simulink model "reduce_spect"  (R2013b) (continued)
== system reduce_spect == (continued)
      mask prompts: storage "Storage medium"; dram_dimm "DRAM dimm"; dram_clock "DRAM clock rate"; nsamples "Number of Samples (2^?)"; data_width "Data width"; offset "Start delay support"; circap "Circular capture support"; value "Extra value capture support"; use_dsp48 "Use DSP48s to implement counters"
      mask help:
      | Standard snap block, but with the following optional extras;
      |  1) Optionally delay the fabric trigger by a user-specified number of valids.
      |  2) Capture continuously after a trigger until a stop pulse is received. Data is captured into BRAM continuously, with old data being overwritten until a stop command is received. Then stop capturing when memory is filled. This enables you to get some data before and some data after the stop pulse. Use the 'tr_en_cnt' register to determine where you are in your vector. 'Addr' register's MSb now indicates 'busy capturing'.
      |  3) Optionally capture a value on an input port to a register at the same time as the first data sample.
      |  4) Implement counters using DSP48Es to save resources.
      mask init: same as reduce_spect/adcsnap1
      mask TypeOptions storage: bram | dram
      mask Callback storage: snapshot_callback();
      mask TypeOptions dram_dimm: 1 | 2 | 3 | 4
      mask TypeOptions dram_clock: 200 | 250 | 266 | 300 | 333
      mask TypeOptions data_width: 8 | 16 | 32 | 64 | 128
  spec1_0 : Subsystem  Tag=xps:bram  AncestorBlock=xps_library/Shared_BRAM  mask{arith_type=Unsigned, addr_width=9, data_width=64, reg_prim_output=on, reg_core_output=on, optimization=Minimum_Area, data_bin_pt=0, init_vals=[0:2^10-1], sample_rate=1}
      mask prompts: arith_type "Output Data Type"; addr_width "Address width"; data_width "Data Width"; reg_prim_output "Register Primitive Output"; reg_core_output "Register Core Output"; data_bin_pt "Data Binary Point"; init_vals "Initial values (simulation only)"; sample_rate "Sample rate"
      mask init:
      | shared_bram_mask;
      mask TypeOptions arith_type: Unsigned | Signed  (2's comp)
      mask TypeOptions data_width: 8 | 16 | 32 | 64 | 128
      mask TypeOptions optimization: Minimum_Area | Low_Power
  spec1_1 : Subsystem  Tag=xps:bram  AncestorBlock=xps_library/Shared_BRAM  mask{arith_type=Unsigned, addr_width=9, data_width=64, reg_prim_output=on, reg_core_output=on, optimization=Minimum_Area, data_bin_pt=0, init_vals=[0:2^10-1], sample_rate=1}
      mask prompts: arith_type "Output Data Type"; addr_width "Address width"; data_width "Data Width"; reg_prim_output "Register Primitive Output"; reg_core_output "Register Core Output"; data_bin_pt "Data Binary Point"; init_vals "Initial values (simulation only)"; sample_rate "Sample rate"
      mask init:
      | shared_bram_mask;
      mask TypeOptions arith_type: Unsigned | Signed  (2's comp)
      mask TypeOptions data_width: 8 | 16 | 32 | 64 | 128
      mask TypeOptions optimization: Minimum_Area | Low_Power
  spec1_2 : Subsystem  Tag=xps:bram  AncestorBlock=xps_library/Shared_BRAM  mask{arith_type=Unsigned, addr_width=9, data_width=64, reg_prim_output=on, reg_core_output=on, optimization=Minimum_Area, data_bin_pt=0, init_vals=[0:2^10-1], sample_rate=1}
      mask prompts: arith_type "Output Data Type"; addr_width "Address width"; data_width "Data Width"; reg_prim_output "Register Primitive Output"; reg_core_output "Register Core Output"; data_bin_pt "Data Binary Point"; init_vals "Initial values (simulation only)"; sample_rate "Sample rate"
      mask init:
      | shared_bram_mask;
      mask TypeOptions arith_type: Unsigned | Signed  (2's comp)
      mask TypeOptions data_width: 8 | 16 | 32 | 64 | 128
      mask TypeOptions optimization: Minimum_Area | Low_Power
  spec1_3 : Subsystem  Tag=xps:bram  AncestorBlock=xps_library/Shared_BRAM  mask{arith_type=Unsigned, addr_width=9, data_width=64, reg_prim_output=on, reg_core_output=on, optimization=Minimum_Area, data_bin_pt=0, init_vals=[0:2^10-1], sample_rate=1}
      mask prompts: arith_type "Output Data Type"; addr_width "Address width"; data_width "Data Width"; reg_prim_output "Register Primitive Output"; reg_core_output "Register Core Output"; data_bin_pt "Data Binary Point"; init_vals "Initial values (simulation only)"; sample_rate "Sample rate"
      mask init:
      | shared_bram_mask;
      mask TypeOptions arith_type: Unsigned | Signed  (2's comp)
      mask TypeOptions data_width: 8 | 16 | 32 | 64 | 128
      mask TypeOptions optimization: Minimum_Area | Low_Power
  spec2_0 : Subsystem  Tag=xps:bram  AncestorBlock=xps_library/Shared_BRAM  mask{arith_type=Unsigned, addr_width=9, data_width=64, reg_prim_output=on, reg_core_output=on, optimization=Minimum_Area, data_bin_pt=0, init_vals=[0:2^10-1], sample_rate=1}
      mask prompts: arith_type "Output Data Type"; addr_width "Address width"; data_width "Data Width"; reg_prim_output "Register Primitive Output"; reg_core_output "Register Core Output"; data_bin_pt "Data Binary Point"; init_vals "Initial values (simulation only)"; sample_rate "Sample rate"
      mask init:
      | shared_bram_mask;
      mask TypeOptions arith_type: Unsigned | Signed  (2's comp)
      mask TypeOptions data_width: 8 | 16 | 32 | 64 | 128
      mask TypeOptions optimization: Minimum_Area | Low_Power
  spec2_1 : Subsystem  Tag=xps:bram  AncestorBlock=xps_library/Shared_BRAM  mask{arith_type=Unsigned, addr_width=9, data_width=64, reg_prim_output=on, reg_core_output=on, optimization=Minimum_Area, data_bin_pt=0, init_vals=[0:2^10-1], sample_rate=1}
      mask prompts: arith_type "Output Data Type"; addr_width "Address width"; data_width "Data Width"; reg_prim_output "Register Primitive Output"; reg_core_output "Register Core Output"; data_bin_pt "Data Binary Point"; init_vals "Initial values (simulation only)"; sample_rate "Sample rate"
      mask init:
      | shared_bram_mask;
      mask TypeOptions arith_type: Unsigned | Signed  (2's comp)
      mask TypeOptions data_width: 8 | 16 | 32 | 64 | 128
      mask TypeOptions optimization: Minimum_Area | Low_Power
  spec2_2 : Subsystem  Tag=xps:bram  AncestorBlock=xps_library/Shared_BRAM  mask{arith_type=Unsigned, addr_width=9, data_width=64, reg_prim_output=on, reg_core_output=on, optimization=Minimum_Area, data_bin_pt=0, init_vals=[0:2^10-1], sample_rate=1}
      mask prompts: arith_type "Output Data Type"; addr_width "Address width"; data_width "Data Width"; reg_prim_output "Register Primitive Output"; reg_core_output "Register Core Output"; data_bin_pt "Data Binary Point"; init_vals "Initial values (simulation only)"; sample_rate "Sample rate"
      mask init:
      | shared_bram_mask;
      mask TypeOptions arith_type: Unsigned | Signed  (2's comp)
      mask TypeOptions data_width: 8 | 16 | 32 | 64 | 128
      mask TypeOptions optimization: Minimum_Area | Low_Power
  spec2_3 : Subsystem  Tag=xps:bram  AncestorBlock=xps_library/Shared_BRAM  mask{arith_type=Unsigned, addr_width=9, data_width=64, reg_prim_output=on, reg_core_output=on, optimization=Minimum_Area, data_bin_pt=0, init_vals=[0:2^10-1], sample_rate=1}
      mask prompts: arith_type "Output Data Type"; addr_width "Address width"; data_width "Data Width"; reg_prim_output "Register Primitive Output"; reg_core_output "Register Core Output"; data_bin_pt "Data Binary Point"; init_vals "Initial values (simulation only)"; sample_rate "Sample rate"
      mask init:
      | shared_bram_mask;
      mask TypeOptions arith_type: Unsigned | Signed  (2's comp)
      mask TypeOptions data_width: 8 | 16 | 32 | 64 | 128
      mask TypeOptions optimization: Minimum_Area | Low_Power
  sync_gen : Subsystem  AncestorBlock=casper_library_misc/sync_gen  mask(sync_gen){sim_acc_len=1, fft_size=2048, fft_simult_inputs=8, pfb_fir_taps=4, reorder_vec=[2 2 9], scale=1}
      mask prompts: sim_acc_len "Simulation Accumulation Length"; fft_size "FFT Size"; fft_simult_inputs "Simultaneous Inputs (FFT)"; pfb_fir_taps "Taps in pfb_fir (use 1 if no pfb_fir)"; reorder_vec "Reorder Orders (e.g. [2 2 2])"
      mask help:
      | This block computes the sync period based on system parameters as
      | described in Casper memo.  Detailed descritions of the block's
      | variables can be found in the 'Block Documentation' section of
      | the CASPER wiki.
      mask init:
      | sync_gen_init(gcb, ...
      |     'sim_acc_len', sim_acc_len, ...
      |     'fft_size', fft_size, ...
      |     'fft_simult_inputs', fft_simult_inputs,...
      |     'pfb_fir_taps', pfb_fir_taps, ...
      |     'reorder_vec', reorder_vec, ...
      |     'scale', scale);
      settings: AttributesFormatString=sim_sync_period=18432
      mask Help:
      | SyncPeriod=LCM(reorder_vec)*(acc_num)*(pfb_fir_len)*(fft_size/fft_simult_inputs)
      | 
      | See CASPER sync pulse memo for more details.
  sync_tree2 : Subsystem
  ten_Gbe_v2 : Subsystem  Tag=xps:tengbe_v2  AncestorBlock=xps_library/ten_Gbe_v2  mask(ten_GbE_v2){flavour=sfp+, slot=0, port_r1=0, port_r2_cx4=0, port_r2_sfpp=0, rx_dist_ram=off, large_frames=on, show_param=on, pre_emph_r2=1.07, pre_emph=3, post_emph_r2=0.18, rxeqmix_r2=7, swing_r2=940, swing=800, fab_en=on, fab_mac=hex2dec('123456780000'), fab_ip=192*(2^24) + 168*(2^16) + 5*(2^8) + 20*(2^0), fab_udp=10000, fab_gate=1, cpu_rx_en=off, cpu_tx_en=on, ttl=hex2dec('FF'), promisc_mode=off, debug_ctr_width=16, txctr=on, txerrctr=on, txerrctr_len=100, txofctr=on, txfullctr=on, txvldctr=on, txsnaplen=16384, rxctr=off, rxerrctr=off, rxerrctr_len=100, rxofctr=off, rxbadctr=off, rxvldctr=off, rxeofctr=off, rxsnaplen=0 - no snap}
      mask prompts: flavour "Mezzanine card flavour"; slot "Card slot"; port_r1 "Port"; port_r2_cx4 "Port"; port_r2_sfpp "Port"; rx_dist_ram "Shallow RX Fifo (Beware overruns!)"; large_frames "Enable Large TX Frames (8k+512)"; show_param "---------------------- Show Implementation Parameters -----------------------------------------------------------------------------------"; pre_emph_r2 "Pre-emphasis (dB)"; pre_emph "Pre-emphasis"; post_emph_r2 "Post-emphasis (dB)"; rxeqmix_r2 "Receive equalisation"; swing_r2 "Driver Swing Control (mVppd)"; swing "Differential swing"; fab_en "Enable fabric on startup"; fab_mac "Fabric MAC Address"; fab_ip "Fabric IP Address"; fab_udp "Fabric UDP Port"; fab_gate "Fabric Gateway"; cpu_rx_en "Enable CPU RX"; cpu_tx_en "Enable CPU TX"; promisc_mode "Promiscuous mode"; debug_ctr_width "Debug counter width"; txctr "TX - core_txctr"; txerrctr "TX packet length error - core_txerrctr"; txerrctr_len "-- expected TX packet length"; txofctr "TX overflow - core_txofctr"; txfullctr "TX full - core_txfullctr"; txvldctr "TX valid cycle - core_txvldctr"; txsnaplen "TX snapshot length"; rxctr "RX - core_rxctr"; rxerrctr "RX packet length error - core_rxerrctr"; rxerrctr_len "-- expected RX packet length"; rxofctr "RX overflow - core_rxofctr"; rxbadctr "RX bad - core_rxbadctr"; rxvldctr "RX valid cycle - core_rxvldctr"; rxeofctr "RX eof - core_rxeofctr"; rxsnaplen "RX snapshot length"
      mask help: This block sends and receives UDP frames (packets). It accepts a 64 bit wide data stream with user-determined frame breaks. The data stream is wrapped in a UDP frame for transmission. Incoming UDP packets are unwrapped and the data presented as a 64 bit wide stream.
      mask init:
      | temp = eval(get_param(gcb, 'debug_ctr_width'));
      | if temp <= 0,
      |     error('Debug counters cannot be ltoet zero.');
      | end
      | tengbe_v2_mask(gcb)
      mask TypeOptions flavour: sfp+ | cx4
      mask Callback flavour: tengbe_v2_callback
      mask TypeOptions slot: 0 | 1
      mask TypeOptions port_r1: 0 | 1 | 2 | 3
      mask TypeOptions port_r2_cx4: 0 | 1 | 2
      mask TypeOptions port_r2_sfpp: 0 | 1 | 2 | 3
      mask Callback show_param: tengbe_v2_callback
      mask TypeOptions pre_emph_r2: 0.15 | 0.3 | 0.45 | 0.61 | 0.74 | 0.91 | 1.07 | 1.25 | 1.36 | 1.55 | 1.74 | 1.94 | 2.11 | 2.32 | 2.54 | 2.77
      mask TypeOptions pre_emph: 0 | 1 | 2 | 3
      mask TypeOptions post_emph_r2: 0.18 | 0.19 | 0.2 | 0.39 | 0.63 | 0.82 | 1.07 | 1.32 | 1.6 | 1.65 | 1.94 | 2.21 | 2.52 | 2.76 | 3.08 | 3.41 | 3.77 | 3.97 | 4.36 | 4.73 | 5.16 | 5.47 | 5.93 | 6.38 | 6.89
      mask TypeOptions rxeqmix_r2: 0 | 1 | 2 | 3 | 4 | 5 | 6 | 7
      mask TypeOptions swing_r2: 110 | 210 | 310 | 400 | 480 | 570 | 660 | 740 | 810 | 880 | 940 | 990 | 1040 | 1080 | 1110 | 1130
      mask TypeOptions swing: 400 | 500 | 600 | 700 | 800
      mask Callback txerrctr:
      | blk = gcb;
      | mask_visibilities = get_param(blk, 'MaskVisibilities');
      | mask_names = get_param(blk, 'MaskNames');
      | if strcmp(get_param(blk, 'txerrctr'), 'on')
      |     mask_visibilities{ismember(mask_names, 'txerrctr_len')} = 'on';
      | else
      |     mask_visibilities{ismember(mask_names, 'txerrctr_len')} = 'off';
      | end
      | set_param(blk, 'MaskVisibilities', mask_visibilities);
      mask TypeOptions txsnaplen: 0 - no snap | 256 | 512 | 1024 | 2048 | 4096 | 8192 | 16384
      mask Callback rxerrctr:
      | blk = gcb;
      | mask_visibilities = get_param(blk, 'MaskVisibilities');
      | mask_names = get_param(blk, 'MaskNames');
      | if strcmp(get_param(blk, 'rxerrctr'), 'on')
      |     mask_visibilities{ismember(mask_names, 'rxerrctr_len')} = 'on';
      | else
      |     mask_visibilities{ismember(mask_names, 'rxerrctr_len')} = 'off';
      | end
      | set_param(blk, 'MaskVisibilities', mask_visibilities);
      mask TypeOptions rxsnaplen: 0 - no snap | 256 | 512 | 1024 | 2048 | 4096 | 8192 | 16384
  test : Subsystem  Tag=xps:bram  AncestorBlock=xps_library/Shared_BRAM  mask{arith_type=Unsigned, addr_width=10, data_width=32, reg_prim_output=off, reg_core_output=off, optimization=Minimum_Area, data_bin_pt=0, init_vals=[0:2^10-1], sample_rate=1}
      mask prompts: arith_type "Output Data Type"; addr_width "Address width"; data_width "Data Width"; reg_prim_output "Register Primitive Output"; reg_core_output "Register Core Output"; data_bin_pt "Data Binary Point"; init_vals "Initial values (simulation only)"; sample_rate "Sample rate"
      mask init:
      | shared_bram_mask;
      mask TypeOptions arith_type: Unsigned | Signed  (2's comp)
      mask TypeOptions data_width: 8 | 16 | 32 | 64 | 128
      mask TypeOptions optimization: Minimum_Area | Low_Power
  theta0 : Subsystem  Tag=xps:sw_reg  AncestorBlock=xps_library/software_register  mask(swreg){io_dir=From Processor, io_delay=0, sample_period=1, names=reg, bitwidths=32, bin_pts=0, arith_types=0, sim_port=off, show_format=on}
      mask prompts: io_dir "I/O direction"; io_delay "I/O delay"; sample_period "Sample period"; names "Bitfield names [msb...lsb]"; bitwidths "Bitfield widths"; bin_pts "Bitfield binary pts"; arith_types "Bitfield types, ufix=0, fix=1, bool=2"; sim_port "Provide sim input/output?"; show_format "Print format string?"
      mask help: A 32-bit software-accessible register. Can be divided into bitfields of varying widths and types using the fields in the block mask.
      mask init:
      | try
      |     swreg_init(gcb)
      | catch ex
      |     disp(sprintf('%s: %s', gcb, ex.message))
      |     errordlg(ex.message)
      | end
      settings: AttributesFormatString=1input:uf32.0 = 32 bits
      mask TypeOptions io_dir: To Processor | From Processor
      mask Callback io_dir:
      | mask_enables = get_param(gcb, 'MaskEnables');
      | mask_names = get_param(gcb, 'MaskNames');
      | if strcmp(get_param(gcb, 'io_dir'), 'To Processor'),
      |     mask_enables{ismember(mask_names, 'sample_period')} = 'off';
      | else
      |     mask_enables{ismember(mask_names, 'sample_period')} = 'on';
      | end
      | set_param(gcb, 'MaskEnables', mask_enables);
  theta1 : Subsystem  Tag=xps:sw_reg  AncestorBlock=xps_library/software_register  mask(swreg){io_dir=From Processor, io_delay=0, sample_period=1, names=reg, bitwidths=32, bin_pts=0, arith_types=0, sim_port=off, show_format=on}
      mask prompts: io_dir "I/O direction"; io_delay "I/O delay"; sample_period "Sample period"; names "Bitfield names [msb...lsb]"; bitwidths "Bitfield widths"; bin_pts "Bitfield binary pts"; arith_types "Bitfield types, ufix=0, fix=1, bool=2"; sim_port "Provide sim input/output?"; show_format "Print format string?"
      mask help: A 32-bit software-accessible register. Can be divided into bitfields of varying widths and types using the fields in the block mask.
      mask init:
      | try
      |     swreg_init(gcb)
      | catch ex
      |     disp(sprintf('%s: %s', gcb, ex.message))
      |     errordlg(ex.message)
      | end
      settings: AttributesFormatString=1input:uf32.0 = 32 bits
      mask TypeOptions io_dir: To Processor | From Processor
      mask Callback io_dir:
      | mask_enables = get_param(gcb, 'MaskEnables');
      | mask_names = get_param(gcb, 'MaskNames');
      | if strcmp(get_param(gcb, 'io_dir'), 'To Processor'),
      |     mask_enables{ismember(mask_names, 'sample_period')} = 'off';
      | else
      |     mask_enables{ismember(mask_names, 'sample_period')} = 'on';
      | end
      | set_param(gcb, 'MaskEnables', mask_enables);
  theta2 : Subsystem  Tag=xps:sw_reg  AncestorBlock=xps_library/software_register  mask(swreg){io_dir=From Processor, io_delay=0, sample_period=1, names=reg, bitwidths=32, bin_pts=0, arith_types=0, sim_port=off, show_format=on}
      mask prompts: io_dir "I/O direction"; io_delay "I/O delay"; sample_period "Sample period"; names "Bitfield names [msb...lsb]"; bitwidths "Bitfield widths"; bin_pts "Bitfield binary pts"; arith_types "Bitfield types, ufix=0, fix=1, bool=2"; sim_port "Provide sim input/output?"; show_format "Print format string?"
      mask help: A 32-bit software-accessible register. Can be divided into bitfields of varying widths and types using the fields in the block mask.
      mask init:
      | try
      |     swreg_init(gcb)
      | catch ex
      |     disp(sprintf('%s: %s', gcb, ex.message))
      |     errordlg(ex.message)
      | end
      settings: AttributesFormatString=1input:uf32.0 = 32 bits
      mask TypeOptions io_dir: To Processor | From Processor
      mask Callback io_dir:
      | mask_enables = get_param(gcb, 'MaskEnables');
      | mask_names = get_param(gcb, 'MaskNames');
      | if strcmp(get_param(gcb, 'io_dir'), 'To Processor'),
      |     mask_enables{ismember(mask_names, 'sample_period')} = 'off';
      | else
      |     mask_enables{ismember(mask_names, 'sample_period')} = 'on';
      | end
      | set_param(gcb, 'MaskEnables', mask_enables);
  theta3 : Subsystem  Tag=xps:sw_reg  AncestorBlock=xps_library/software_register  mask(swreg){io_dir=From Processor, io_delay=0, sample_period=1, names=reg, bitwidths=32, bin_pts=0, arith_types=0, sim_port=off, show_format=on}
      mask prompts: io_dir "I/O direction"; io_delay "I/O delay"; sample_period "Sample period"; names "Bitfield names [msb...lsb]"; bitwidths "Bitfield widths"; bin_pts "Bitfield binary pts"; arith_types "Bitfield types, ufix=0, fix=1, bool=2"; sim_port "Provide sim input/output?"; show_format "Print format string?"
      mask help: A 32-bit software-accessible register. Can be divided into bitfields of varying widths and types using the fields in the block mask.
      mask init:
      | try
      |     swreg_init(gcb)
      | catch ex
      |     disp(sprintf('%s: %s', gcb, ex.message))
      |     errordlg(ex.message)
      | end
      settings: AttributesFormatString=1input:uf32.0 = 32 bits
      mask TypeOptions io_dir: To Processor | From Processor
      mask Callback io_dir:
      | mask_enables = get_param(gcb, 'MaskEnables');
      | mask_names = get_param(gcb, 'MaskNames');
      | if strcmp(get_param(gcb, 'io_dir'), 'To Processor'),
      |     mask_enables{ismember(mask_names, 'sample_period')} = 'off';
      | else
      |     mask_enables{ismember(mask_names, 'sample_period')} = 'on';
      | end
      | set_param(gcb, 'MaskEnables', mask_enables);
  wires:
  cnt_rst/1(in_reg) -> pipeline5/1(d)
  pipeline5/1(q) -> Slice3/1, Slice/1
  Slice/1 -> [cnt_rst]
  acc_len/1(in_reg) -> pipeline6/1(d)
  pipeline6/1(q) -> [acc_len]
  sync_gen/1(sync_out) -> pipeline143/1(d)
  pipeline143/1(q) -> [sync_gen]
  [acc_len0] -> acc_cntrl2/1(acc_len)
  [cnt_rst] -> acc_cntrl2/3(rst)
  [sd0] -> acc_cntrl2/2(sync)
  [m0d0] -> Delay26/1
  [m0e0] -> Delay27/1
  Delay26/1 -> Delay28/1
  acc_cntrl2/1(new_acc) -> Delay29/1
  Delay27/1 -> Delay30/1
  Delay29/1 -> pipeline9/1(d), simple_bram_vacc/1(new_acc), Delay14/1
  Delay30/1 -> simple_bram_vacc/3(val_in)
  Register/1 -> [frb_detector_0]
  [rst_detect] -> Register/2
  Relational/1 -> Register/3, Register/1
  Accumulator1/1 -> Reinterpret3/1
  Counter2/1 -> ACC0/1(addr)
  ACC0/1(data_out) -> Terminator22/1
  theta0/1(in_reg) -> Convert1/1
  Convert1/1 -> Relational/1
  acc_len1/1(in_reg) -> pipeline30/1(d)
  pipeline30/1(q) -> [acc_len1]
  gain/1(in_reg) -> pipeline31/1(d)
  pipeline31/1(q) -> [gain]
  [sync_gen] -> pipeline41/1(d)
  Delay37/1 -> Delay57/1
  Delay35/1 -> power4/1
  pipeline41/1(q) -> pfb_fir_real/1(sync)
  asiaa_adc5g/17(sync_out) -> Terminator6/1
  conv_bus1/1(q0) -> pipeline301/1
  conv_bus1/2(q1) -> pipeline349/1
  conv_bus1/3(q2) -> pipeline399/1
  conv_bus1/4(q3) -> pipeline400/1
  conv_bus1/5(q4) -> pipeline401/1
  conv_bus1/6(q5) -> pipeline402/1
  conv_bus1/7(q6) -> pipeline300/1
  conv_bus1/8(q7) -> pipeline299/1
  pipeline34/1(q) -> pfb_fir_real/4(pol1_in3)
  pipeline37/1(q) -> pfb_fir_real/6(pol1_in5)
  pipeline32/1(q) -> pfb_fir_real/2(pol1_in1)
  pipeline33/1(q) -> pfb_fir_real/3(pol1_in2)
  pipeline36/1(q) -> pfb_fir_real/5(pol1_in4)
  pipeline38/1(q) -> pfb_fir_real/7(pol1_in6)
  pipeline39/1(q) -> pfb_fir_real/8(pol1_in7)
  pipeline40/1(q) -> pfb_fir_real/9(pol1_in8)
  Delay62/1 -> fft_wideband_real/1(sync)
  pfb_fir_real/1(sync_out) -> Delay62/1
  Constant/1 -> fft_wideband_real/2(shift)
  pfb_fir_real/2(pol1_out1) -> Delay32/1
  pfb_fir_real/3(pol1_out2) -> Delay61/1
  pfb_fir_real/4(pol1_out3) -> Delay63/1
  pfb_fir_real/5(pol1_out4) -> Delay79/1
  pfb_fir_real/6(pol1_out5) -> Delay34/1
  pfb_fir_real/7(pol1_out6) -> Delay38/1
  pfb_fir_real/8(pol1_out7) -> Delay39/1
  pfb_fir_real/9(pol1_out8) -> Delay48/1
  Delay48/1 -> fft_wideband_real/10(in07)
  Delay34/1 -> fft_wideband_real/7(in04)
  Delay79/1 -> fft_wideband_real/6(in03)
  Delay38/1 -> fft_wideband_real/8(in05)
  Delay63/1 -> fft_wideband_real/5(in02)
  Delay32/1 -> fft_wideband_real/3(in00)
  Delay39/1 -> fft_wideband_real/9(in06)
  Delay61/1 -> fft_wideband_real/4(in01)
  fft_wideband_real/6(of) -> Terminator7/1
  fft_wideband_real/1(sync_out) -> Delay37/1
  Delay58/1 -> power5/1
  Delay59/1 -> power6/1
  Delay60/1 -> power7/1
  fft_wideband_real/2(out00) -> Delay35/1
  fft_wideband_real/3(out01) -> Delay58/1
  fft_wideband_real/4(out02) -> Delay59/1
  fft_wideband_real/5(out03) -> Delay60/1
  bus_create1/1(bus_out) -> pipeline599/1(d)
  Constant123/1 -> adcsnap1/3(trig), adcsnap1/2(we)
  pipeline599/1(q) -> adcsnap1/1(din)
  Delay57/1 -> Delay68/1
  power7/1 -> Delay71/1
  power5/1 -> Delay69/1
  power4/1 -> Delay67/1
  power6/1 -> Delay70/1
  Delay71/1 -> pipeline347/1(d), Delay65/1
  Delay69/1 -> pipeline345/1(d), Delay73/1
  Delay66/1 -> acc_cntrl11/2(sync)
  [acc_len] -> acc_cntrl11/1(acc_len)
  [cnt_rst] -> acc_cntrl11/3(rst)
  Delay72/1 -> pipeline304/1(d)
  pipeline315/1(q) -> simple_bram_vacc46/1(new_acc)
  pipeline311/1(q) -> simple_bram_vacc46/2(din)
  pipeline314/1(q) -> pipeline105/1(d)
  acc_cntrl11/1(new_acc) -> pipeline398/1(d)
  pipeline304/1(q) -> pipeline394/1(d)
  simple_bram_vacc46/1(dout) -> pipeline97/1(d)
  spec1_0/1(data_out) -> Terminator46/1
  simple_bram_vacc46/2(valid) -> pipeline98/1(d)
  pipeline305/1(q) -> simple_bram_vacc43/1(new_acc)
  pipeline303/1(q) -> simple_bram_vacc43/2(din)
  simple_bram_vacc43/1(dout) -> pipeline99/1(d)
  spec1_1/1(data_out) -> Terminator43/1
  simple_bram_vacc43/2(valid) -> pipeline100/1(d)
  pipeline309/1(q) -> simple_bram_vacc45/1(new_acc)
  pipeline308/1(q) -> simple_bram_vacc45/2(din)
  simple_bram_vacc45/1(dout) -> pipeline101/1(d)
  spec1_2/1(data_out) -> Terminator45/1
  simple_bram_vacc45/2(valid) -> pipeline102/1(d)
  pipeline307/1(q) -> simple_bram_vacc44/1(new_acc)
  pipeline306/1(q) -> simple_bram_vacc44/2(din)
  simple_bram_vacc44/1(dout) -> pipeline103/1(d)
  spec1_3/1(data_out) -> Terminator44/1
  simple_bram_vacc44/2(valid) -> pipeline104/1(d)
  Counter22/1 -> spec1_0/1(addr), spec1_1/1(addr), spec1_2/1(addr), spec1_3/1(addr)
  Delay73/1 -> pipeline310/1(d)
  pipeline310/1(q) -> pipeline395/1(d)
  Delay64/1 -> pipeline312/1(d)
  pipeline312/1(q) -> pipeline396/1(d)
  Delay65/1 -> pipeline313/1(d)
  pipeline313/1(q) -> pipeline397/1(d)
  Delay68/1 -> pipeline351/1(d), Delay66/1
  Delay67/1 -> pipeline348/1(d), Delay72/1
  Delay70/1 -> pipeline346/1(d), Delay64/1
  pipeline397/1(q) -> pipeline306/1(d)
  pipeline394/1(q) -> pipeline311/1(d)
  pipeline396/1(q) -> pipeline308/1(d)
  pipeline395/1(q) -> pipeline303/1(d)
  pipeline398/1(q) -> pipeline314/1(d), pipeline307/1(d), pipeline309/1(d), pipeline305/1(d), pipeline315/1(d)
  pipeline298/1 -> bus_expand/1(bus_in)
  conv_bus1/9(q8) -> pipeline298/1, [adc1_a_red], [adc0_a_red]
  pipeline299/1 -> pipeline40/1(d), bus_create1/8(in8)
  pipeline300/1 -> pipeline39/1(d), bus_create1/7(in7)
  pipeline301/1 -> pipeline32/1(d), bus_create1/1(in1)
  pipeline349/1 -> pipeline33/1(d), bus_create1/2(in2)
  pipeline399/1 -> pipeline34/1(d), bus_create1/3(in3)
  pipeline400/1 -> pipeline36/1(d), bus_create1/4(in4)
  pipeline401/1 -> pipeline37/1(d), bus_create1/5(in5)
  pipeline402/1 -> pipeline38/1(d), bus_create1/6(in6)
  pipeline351/1(q) -> adder_tree1/1(sync)
  pipeline348/1(q) -> adder_tree1/2(din1)
  pipeline345/1(q) -> adder_tree1/3(din2)
  pipeline346/1(q) -> adder_tree1/4(din3)
  pipeline347/1(q) -> adder_tree1/5(din4)
  Counter4/1 -> Slice8/1, Slice7/1, Slice6/1
  Slice6/1 -> Logical1/2
  Slice7/1 -> Logical1/3
  Subsystem3/1(Out1) -> Delay7/1
  [gain] -> Mult5/2
  Mult5/1 -> pipeline15/1(d)
  pipeline49/1(q) -> pipeline16/1(d)
  Delay82/1 -> Delay/1
  Slice8/1 -> Logical1/1
  asiaa_adc5g/6(a5) -> conv_bus1/7(i5)
  asiaa_adc5g/4(a3) -> conv_bus1/5(i3)
  asiaa_adc5g/1(a0) -> conv_bus1/2(i0)
  asiaa_adc5g/5(a4) -> conv_bus1/6(i4)
  asiaa_adc5g/2(a1) -> conv_bus1/3(i1)
  asiaa_adc5g/3(a2) -> conv_bus1/4(i2)
  asiaa_adc5g/7(a6) -> conv_bus1/8(i6)
  asiaa_adc5g/8(a7) -> conv_bus1/9(i7)
  asiaa_adc5g/10(c1) -> conv_bus2/3(i1)
  asiaa_adc5g/11(c2) -> conv_bus2/4(i2)
  asiaa_adc5g/12(c3) -> conv_bus2/5(i3)
  asiaa_adc5g/13(c4) -> conv_bus2/6(i4)
  asiaa_adc5g/14(c5) -> conv_bus2/7(i5)
  asiaa_adc5g/15(c6) -> conv_bus2/8(i6)
  asiaa_adc5g/16(c7) -> conv_bus2/9(i7)
  Dedispersor_v2/1(Output) -> Delay4/1
  simple_bram_vacc/2(valid) -> Logical/2, pipeline/1, Counter/2
  simple_bram_vacc/1(dout) -> pipeline1/1
  Mux/1 -> Convert/1, Dedispersor_v2/1(Data)
  Logical/1 -> pipeline2/1
  pipeline2/1 -> Mux/1
  Counter1/1 -> small_spec2/1(addr)
  pipeline3/1(q) -> Reinterpret1/1
  pipeline4/1(q) -> Counter1/1
  pipeline7/1(q) -> small_spec2/3(we), Counter1/2
  Reinterpret1/1 -> small_spec2/2(data_in)
  pipeline8/1 -> pipeline4/1(d)
  Convert/1 -> pipeline3/1(d)
  Reinterpret/1 -> Convert2/1
  Delay/1 -> Delay5/1
  Delay1/1 -> Delay9/1
  Counter3/1 -> test/1(addr)
  Convert2/1 -> Delay2/1
  pipeline50/1(q) -> pipeline13/1(d)
  Delay81/1 -> Delay1/1
  Reinterpret2/1 -> test/2(data_in)
  Convert3/1 -> Reinterpret2/1
  Delay80/1 -> Reinterpret/1
  Counter/1 -> Relational1/2
  Relational1/1 -> Logical/1
  Constant4/1 -> Relational1/1
  adder_tree1/2(dout) -> Subsystem3/1(In1)
  gain_adc/1(in_reg) -> pipeline14/1(d)
  pipeline14/1(q) -> [gain_adc]
  [gain_adc] -> conv_bus1/1(gain)
  Constant6/1 -> snapshot/2(we), snapshot/3(trig)
  bus_expand/1(msb_out8) -> Convert4/1
  bus_expand/2(out7) -> Convert5/1
  bus_expand/3(out6) -> Convert6/1
  bus_expand/4(out5) -> Convert7/1
  bus_expand/5(out4) -> Convert8/1
  bus_expand/6(out3) -> Convert9/1
  bus_expand/7(out2) -> Convert10/1
  bus_expand/8(lsb_out1) -> Convert11/1
  Convert11/1 -> bus_create2/8(in8)
  Convert10/1 -> bus_create2/7(in7)
  Convert9/1 -> bus_create2/6(in6)
  Convert8/1 -> bus_create2/5(in5)
  Convert7/1 -> bus_create2/4(in4)
  Convert6/1 -> bus_create2/3(in3)
  Convert5/1 -> bus_create2/2(in2)
  Convert4/1 -> bus_create2/1(in1)
  bus_create2/1(bus_out) -> snapshot/1(din)
  pipeline9/1(q) -> pipeline8/1
  Constant1/1 -> Mux/3
  pipeline1/1 -> Mux/2
  Logical1/1 -> Delay6/1
  adder_tree1/1(sync_out) -> Delay8/1, Counter4/1
  Delay4/1 -> Accumulator1/1
  Delay6/1 -> Delay81/1
  Delay7/1 -> Delay80/1
  Delay8/1 -> Delay82/1
  Delay28/1 -> simple_bram_vacc/2(din)
  Delay2/1 -> Mult5/1
  Delay5/1 -> pipeline50/1(d)
  Delay9/1 -> pipeline49/1(d)
  Delay10/1 -> test/3(we), Counter3/2
  Delay11/1 -> Convert3/1
  Delay12/1 -> Counter3/1
  pipeline/1 -> pipeline7/1(d), Delay3/1, Dedispersor_v2/2(Valid)
  pipeline13/1(q) -> Delay12/1, pipeline17/1(d)
  pipeline15/1(q) -> pipeline18/1(d), Delay11/1
  pipeline16/1(q) -> Delay10/1, pipeline19/1(d)
  pipeline17/1(q) -> sync_tree2/1(In1)
  pipeline18/1(q) -> m0_tree2/1(In1)
  pipeline19/1(q) -> m0_en1/1(In1)
  Delay3/1 -> Delay13/1
  Counter6/1 -> Slice1/1
  Slice1/1 -> edge_detect/1, Inverter/1
  Inverter/1 -> Logical2/1
  Logical2/1 -> Accumulator1/3, Counter6/2
  edge_detect/1 -> ACC0/3(we), Counter2/1
  Delay13/1 -> Logical2/2
  Delay14/1 -> pipeline12/1, Counter/1
  pipeline12/1 -> Delay15/1
  Delay15/1 -> Accumulator1/2, Counter6/1
  [acc_len2] -> acc_cntrl1/1(acc_len)
  [cnt_rst] -> acc_cntrl1/3(rst)
  [sd1] -> acc_cntrl1/2(sync)
  [m0d1] -> Delay19/1
  [m0e1] -> Delay20/1
  Delay19/1 -> Delay21/1
  acc_cntrl1/1(new_acc) -> Delay22/1
  Delay20/1 -> Delay24/1
  Delay22/1 -> simple_bram_vacc2/1(new_acc), Delay17/1
  Delay24/1 -> simple_bram_vacc2/3(val_in)
  Register1/1 -> [frb_detector_2]
  [rst_detect] -> Register1/2
  Relational2/1 -> Register1/3, Register1/1
  Accumulator2/1 -> Reinterpret6/1
  Counter9/1 -> ACC2/1(addr)
  ACC2/1(data_out) -> Terminator1/1
  theta2/1(in_reg) -> Convert13/1
  Convert13/1 -> Relational2/1
  Dedispersor_v1/1(Output) -> Delay25/1
  simple_bram_vacc2/2(valid) -> Logical3/2, pipeline20/1, Counter7/2
  simple_bram_vacc2/1(dout) -> pipeline21/1
  Mux1/1 -> Dedispersor_v1/1(Data)
  Logical3/1 -> pipeline23/1
  pipeline23/1 -> Mux1/1
  Counter7/1 -> Relational3/2
  Relational3/1 -> Logical3/1
  Constant3/1 -> Relational3/1
  Constant2/1 -> Mux1/3
  pipeline21/1 -> Mux1/2
  Delay25/1 -> Accumulator2/1
  Delay21/1 -> simple_bram_vacc2/2(din)
  pipeline20/1 -> Delay23/1, Dedispersor_v1/2(Valid)
  Delay23/1 -> Delay16/1
  Counter10/1 -> Slice2/1
  Slice2/1 -> edge_detect1/1, Inverter1/1
  Inverter1/1 -> Logical4/1
  Logical4/1 -> Accumulator2/3, Counter10/2
  edge_detect1/1 -> ACC2/3(we), Counter9/1
  Delay16/1 -> Logical4/2
  Delay17/1 -> pipeline22/1, Counter7/1
  pipeline22/1 -> Delay18/1
  Delay18/1 -> Accumulator2/2, Counter10/1
  [acc_len3] -> acc_cntrl4/1(acc_len)
  [cnt_rst] -> acc_cntrl4/3(rst)
  [sd2] -> acc_cntrl4/2(sync)
  [m0d2] -> Delay40/1
  [m0e2] -> Delay41/1
  Delay40/1 -> Delay42/1
  acc_cntrl4/1(new_acc) -> Delay43/1
  Delay41/1 -> Delay45/1
  Delay43/1 -> simple_bram_vacc3/1(new_acc), Delay33/1
  Delay45/1 -> simple_bram_vacc3/3(val_in)
  Register2/1 -> [frb_detector_3]
  [rst_detect] -> Register2/2
  Relational4/1 -> Register2/3, Register2/1
  Accumulator3/1 -> Reinterpret7/1
  Counter12/1 -> ACC3/1(addr)
  ACC3/1(data_out) -> Terminator2/1
  theta3/1(in_reg) -> Convert12/1
  Convert12/1 -> Relational4/1
  Dedispersor_v3/1(Output) -> Delay46/1
  simple_bram_vacc3/2(valid) -> Logical5/2, pipeline24/1, Counter11/2
  simple_bram_vacc3/1(dout) -> pipeline25/1
  Mux2/1 -> Dedispersor_v3/1(Data)
  Logical5/1 -> pipeline27/1
  pipeline27/1 -> Mux2/1
  Counter11/1 -> Relational5/2
  Relational5/1 -> Logical5/1
  Constant7/1 -> Relational5/1
  Constant5/1 -> Mux2/3
  pipeline25/1 -> Mux2/2
  Delay46/1 -> Accumulator3/1
  Delay42/1 -> simple_bram_vacc3/2(din)
  pipeline24/1 -> Delay44/1, Dedispersor_v3/2(Valid)
  Delay44/1 -> Delay31/1
  Counter8/1 -> Slice9/1
  Slice9/1 -> edge_detect2/1, Inverter2/1
  Inverter2/1 -> Logical6/1
  Logical6/1 -> Accumulator3/3, Counter8/2
  edge_detect2/1 -> ACC3/3(we), Counter12/1
  Delay31/1 -> Logical6/2
  Delay33/1 -> pipeline26/1, Counter11/1
  pipeline26/1 -> Delay36/1
  Delay36/1 -> Accumulator3/2, Counter8/1
  acc_len2/1(in_reg) -> pipeline28/1(d)
  pipeline28/1(q) -> [acc_len2]
  acc_len3/1(in_reg) -> pipeline29/1(d)
  pipeline29/1(q) -> [acc_len3]
  [rst] -> pipeline421/1
  [rst] -> pipeline422/1
  [gbe_data] -> pipeline423/1
  [gbe_valid] -> pipeline424/1
  [gbe_eof] -> pipeline430/1
  Delay91/1 -> pipeline428/1
  one_GbE/1 -> Terminator59/1
  one_GbE/2 -> Terminator60/1
  one_GbE/3 -> Terminator61/1
  one_GbE/4 -> Terminator53/1
  one_GbE/5 -> Terminator54/1
  one_GbE/6 -> Terminator55/1
  one_GbE/7 -> Terminator56/1
  one_GbE/8 -> Terminator57/1
  one_GbE/9 -> Terminator58/1
  one_GbE/10 -> Terminator51/1
  one_GbE/11 -> Terminator52/1
  Concat/1 -> pipeline406/1(d)
  ring/1(dout) -> pipeline403/1
  ring/2(eof) -> pipeline404/1
  ring/3(val) -> pipeline405/1
  pipeline407/1 -> Concat/1
  pipeline408/1 -> Concat/2
  pipeline409/1 -> Concat/3
  pipeline406/1(q) -> ring/2(data_in)
  [adc0_a_red] -> pipeline407/1
  [adc0_c_red] -> pipeline408/1
  [adc1_a_red] -> pipeline409/1
  gbe_dest_port/1(in_reg) -> Delay92/1
  gbe_dest_ip/1(in_reg) -> Delay91/1
  control1/1(in_reg) -> Slice12/1, Slice11/1, Slice10/1
  Slice10/1 -> Delay47/1
  Slice11/1 -> Delay49/1
  Delay47/1 -> [write]
  Delay49/1 -> [rst]
  Slice12/1 -> Delay50/1
  Delay50/1 -> [allow_detect]
  [detection] -> Delay93/1
  [write] -> Logical24/2
  Logical23/1 -> Logical24/1
  [allow_detect] -> Inverter3/1
  Logical24/1 -> gpio1/1, ring/1(write_en)
  Inverter3/1 -> Logical23/1
  pipeline403/1 -> [gbe_data]
  pipeline404/1 -> [gbe_eof]
  pipeline405/1 -> [gbe_valid]
  Delay93/1 -> Logical23/2
  pipeline421/1 -> one_GbE/1
  pipeline422/1 -> one_GbE/2
  pipeline423/1 -> one_GbE/3
  pipeline424/1 -> one_GbE/4
  pipeline428/1 -> one_GbE/5
  pipeline429/1 -> one_GbE/6
  pipeline430/1 -> one_GbE/7
  [frb_detector_1] -> pipeline109/1
  [frb_detector_0] -> pipeline108/1
  pipeline111/1 -> Logical12/4
  pipeline108/1 -> Logical12/1
  pipeline109/1 -> Logical12/2
  pipeline110/1 -> Logical12/3
  [frb_detector_3] -> pipeline111/1
  [frb_detector_2] -> pipeline110/1
  Logical12/1 -> pipeline119/1
  pipeline119/1 -> Convert15/1, Register3/1, Register3/3
  Register3/1 -> [detection]
  [rst_detect] -> Register3/2
  Slice3/1 -> pipeline54/1(d)
  Delay92/1 -> pipeline429/1
  [acc_len1] -> acc_cntrl5/1(acc_len)
  [cnt_rst] -> acc_cntrl5/3(rst)
  [sd2] -> acc_cntrl5/2(sync)
  [m0d2] -> Delay54/1
  [m0e2] -> Delay55/1
  Delay54/1 -> Delay56/1
  acc_cntrl5/1(new_acc) -> Delay74/1
  Delay55/1 -> Delay76/1
  Delay74/1 -> simple_bram_vacc4/1(new_acc), Delay52/1
  Delay76/1 -> simple_bram_vacc4/3(val_in)
  Register4/1 -> [frb_detector_1]
  [rst_detect] -> Register4/2
  Relational6/1 -> Register4/3, Register4/1
  Accumulator4/1 -> Reinterpret5/1
  Counter14/1 -> ACC1/1(addr)
  ACC1/1(data_out) -> Terminator3/1
  theta1/1(in_reg) -> Convert14/1
  Convert14/1 -> Relational6/1
  Dedispersor_v4/1(Output) -> Delay77/1
  simple_bram_vacc4/2(valid) -> Logical7/2, pipeline35/1, Counter13/2
  simple_bram_vacc4/1(dout) -> pipeline45/1
  Mux3/1 -> Dedispersor_v4/1(Data)
  Logical7/1 -> pipeline52/1
  pipeline52/1 -> Mux3/1
  Counter13/1 -> Relational7/2
  Relational7/1 -> Logical7/1
  Constant9/1 -> Relational7/1
  Constant8/1 -> Mux3/3
  pipeline45/1 -> Mux3/2
  Delay77/1 -> Accumulator4/1
  Delay56/1 -> simple_bram_vacc4/2(din)
  pipeline35/1 -> Delay75/1, Dedispersor_v4/2(Valid)
  Delay75/1 -> Delay51/1
  Counter15/1 -> Slice4/1
  Slice4/1 -> edge_detect3/1, Inverter4/1
  Inverter4/1 -> Logical8/1
  Logical8/1 -> Accumulator4/3, Counter15/2
  edge_detect3/1 -> ACC1/3(we), Counter14/1
  Delay51/1 -> Logical8/2
  Delay52/1 -> pipeline51/1, Counter13/1
  pipeline51/1 -> Delay53/1
  Delay53/1 -> Accumulator4/2, Counter15/1
  acc_len0/1(in_reg) -> pipeline53/1(d)
  pipeline53/1(q) -> [acc_len0]
  Constant105/1 -> one_GbE/8
  Reinterpret3/1 -> ACC0/2(data_in), Relational/2
  Reinterpret5/1 -> ACC1/2(data_in), Relational6/2
  Reinterpret6/1 -> ACC2/2(data_in), Relational2/2
  Reinterpret7/1 -> ACC3/2(data_in), Relational4/2
  Convert15/1 -> frb_detector/1(out_reg)
  pipeline54/1(q) -> [rst_detect]
  Black Box/3 -> Subsystem/1(data)
  Black Box/2 -> Convert16/1
  Convert16/1 -> Subsystem/2(en)
  gpio3/1 -> Black Box/4
  gps_ctrl/1(in_reg) -> Slice14/1, Slice13/1, Slice5/1
  Slice5/1 -> Convert17/1, Black Box/1
  Slice13/1 -> Black Box/2
  Slice14/1 -> Black Box/3
  gpio6/1 -> Delay78/1
  Black Box/5 -> gpio4/1
  Black Box/1 -> Terminator/1
  Black Box/4 -> Terminator4/1
  Convert17/1 -> Subsystem/3(rst)
  gps_ddmmyy/1(in_reg) -> Subsystem/5(ddmmyy)
  Subsystem/1(secs) -> Concat1/2, sec/1(out_reg)
  Subsystem/4(debug) -> sec_debug/1(out_reg)
  Subsystem/3(pps_debug) -> gpio5/1
  Subsystem/2(subsec) -> Concat1/3
  Concat1/1 -> [time]
  Delay78/1 -> Subsystem/4(pps)
  Subsystem1/1(Out1) -> Concat1/1
  [sync_gen] -> pipeline63/1(d)
  Delay86/1 -> Delay90/1
  Delay85/1 -> power1/1
  pipeline63/1(q) -> pfb_fir_real1/1(sync)
  conv_bus2/1(q0) -> pipeline42/1
  conv_bus2/2(q1) -> pipeline47/1
  conv_bus2/3(q2) -> pipeline58/1
  conv_bus2/4(q3) -> pipeline60/1
  conv_bus2/5(q4) -> pipeline61/1
  conv_bus2/6(q5) -> pipeline62/1
  conv_bus2/7(q6) -> pipeline11/1
  conv_bus2/8(q7) -> pipeline10/1
  pipeline46/1(q) -> pfb_fir_real1/4(pol1_in3)
  pipeline55/1(q) -> pfb_fir_real1/6(pol1_in5)
  pipeline43/1(q) -> pfb_fir_real1/2(pol1_in1)
  pipeline44/1(q) -> pfb_fir_real1/3(pol1_in2)
  pipeline48/1(q) -> pfb_fir_real1/5(pol1_in4)
  pipeline56/1(q) -> pfb_fir_real1/7(pol1_in6)
  pipeline57/1(q) -> pfb_fir_real1/8(pol1_in7)
  pipeline59/1(q) -> pfb_fir_real1/9(pol1_in8)
  Delay98/1 -> fft_wideband_real1/1(sync)
  pfb_fir_real1/1(sync_out) -> Delay98/1
  Constant10/1 -> fft_wideband_real1/2(shift)
  pfb_fir_real1/2(pol1_out1) -> Delay83/1
  pfb_fir_real1/3(pol1_out2) -> Delay97/1
  pfb_fir_real1/4(pol1_out3) -> Delay99/1
  pfb_fir_real1/5(pol1_out4) -> Delay100/1
  pfb_fir_real1/6(pol1_out5) -> Delay84/1
  pfb_fir_real1/7(pol1_out6) -> Delay87/1
  pfb_fir_real1/8(pol1_out7) -> Delay88/1
  pfb_fir_real1/9(pol1_out8) -> Delay89/1
  Delay89/1 -> fft_wideband_real1/10(in07)
  Delay84/1 -> fft_wideband_real1/7(in04)
  Delay100/1 -> fft_wideband_real1/6(in03)
  Delay87/1 -> fft_wideband_real1/8(in05)
  Delay99/1 -> fft_wideband_real1/5(in02)
  Delay83/1 -> fft_wideband_real1/3(in00)
  Delay88/1 -> fft_wideband_real1/9(in06)
  Delay97/1 -> fft_wideband_real1/4(in01)
  fft_wideband_real1/6(of) -> Terminator5/1
  fft_wideband_real1/1(sync_out) -> Delay86/1
  Delay94/1 -> power2/1
  Delay95/1 -> power3/1
  Delay96/1 -> power8/1
  fft_wideband_real1/2(out00) -> Delay85/1
  fft_wideband_real1/3(out01) -> Delay94/1
  fft_wideband_real1/4(out02) -> Delay95/1
  fft_wideband_real1/5(out03) -> Delay96/1
  bus_create3/1(bus_out) -> pipeline64/1(d)
  Constant11/1 -> adcsnap2/3(trig), adcsnap2/2(we)
  pipeline64/1(q) -> adcsnap2/1(din)
  Delay90/1 -> Delay106/1
  power8/1 -> Delay110/1
  power2/1 -> Delay108/1
  power1/1 -> Delay107/1
  power3/1 -> Delay109/1
  conv_bus2/9(q8) -> [adc0_c_red]
  pipeline10/1 -> pipeline59/1(d), bus_create3/8(in8)
  pipeline11/1 -> pipeline57/1(d), bus_create3/7(in7)
  pipeline42/1 -> pipeline43/1(d), bus_create3/1(in1)
  pipeline47/1 -> pipeline44/1(d), bus_create3/2(in2)
  pipeline58/1 -> pipeline46/1(d), bus_create3/3(in3)
  pipeline60/1 -> pipeline48/1(d), bus_create3/4(in4)
  pipeline61/1 -> pipeline55/1(d), bus_create3/5(in5)
  pipeline62/1 -> pipeline56/1(d), bus_create3/6(in6)
  [gain_adc] -> conv_bus2/1(gain)
  asiaa_adc5g/9(c0) -> conv_bus2/2(i0)
  Delay103/1 -> acc_cntrl3/2(sync)
  [acc_len] -> acc_cntrl3/1(acc_len)
  [cnt_rst] -> acc_cntrl3/3(rst)
  Delay104/1 -> pipeline66/1(d)
  pipeline77/1(q) -> simple_bram_vacc7/1(new_acc)
  pipeline73/1(q) -> simple_bram_vacc7/2(din)
  pipeline76/1(q) -> pipeline88/1(d)
  acc_cntrl3/1(new_acc) -> pipeline82/1(d)
  pipeline66/1(q) -> pipeline78/1(d)
  simple_bram_vacc7/1(dout) -> pipeline89/1(d)
  spec2_0/1(data_out) -> Terminator11/1
  simple_bram_vacc7/2(valid) -> pipeline90/1(d)
  pipeline67/1(q) -> simple_bram_vacc1/1(new_acc)
  pipeline65/1(q) -> simple_bram_vacc1/2(din)
  simple_bram_vacc1/1(dout) -> pipeline91/1(d)
  spec2_1/1(data_out) -> Terminator8/1
  simple_bram_vacc1/2(valid) -> pipeline92/1(d)
  pipeline71/1(q) -> simple_bram_vacc6/1(new_acc)
  pipeline70/1(q) -> simple_bram_vacc6/2(din)
  simple_bram_vacc6/1(dout) -> pipeline93/1(d)
  spec2_2/1(data_out) -> Terminator10/1
  simple_bram_vacc6/2(valid) -> pipeline94/1(d)
  pipeline69/1(q) -> simple_bram_vacc5/1(new_acc)
  pipeline68/1(q) -> simple_bram_vacc5/2(din)
  simple_bram_vacc5/1(dout) -> pipeline95/1(d)
  spec2_3/1(data_out) -> Terminator9/1
  simple_bram_vacc5/2(valid) -> pipeline96/1(d)
  Counter5/1 -> spec2_1/1(addr), spec2_2/1(addr), spec2_3/1(addr), spec2_0/1(addr)
  Delay105/1 -> pipeline72/1(d)
  pipeline72/1(q) -> pipeline79/1(d)
  Delay101/1 -> pipeline74/1(d)
  pipeline74/1(q) -> pipeline80/1(d)
  Delay102/1 -> pipeline75/1(d)
  pipeline75/1(q) -> pipeline81/1(d)
  Reinterpret4/1 -> spec2_0/2(data_in)
  Reinterpret8/1 -> spec2_1/2(data_in)
  Reinterpret9/1 -> spec2_2/2(data_in)
  Reinterpret10/1 -> spec2_3/2(data_in)
  pipeline81/1(q) -> pipeline68/1(d)
  pipeline78/1(q) -> pipeline73/1(d)
  pipeline80/1(q) -> pipeline70/1(d)
  pipeline79/1(q) -> pipeline65/1(d)
  pipeline82/1(q) -> pipeline76/1(d), pipeline69/1(d), pipeline71/1(d), pipeline67/1(d), pipeline77/1(d)
  Reinterpret40/1 -> spec1_0/2(data_in)
  Reinterpret41/1 -> spec1_1/2(data_in)
  Reinterpret42/1 -> spec1_2/2(data_in)
  Reinterpret43/1 -> spec1_3/2(data_in)
  pipeline555/1 -> bus_create8/1(in1)
  pipeline556/1 -> bus_create8/2(in2)
  pipeline558/1 -> bus_create8/3(in3)
  pipeline559/1 -> bus_create8/4(in4)
  Convert20/1 -> pipeline555/1
  Convert21/1 -> pipeline556/1
  Convert18/1 -> pipeline558/1
  Convert19/1 -> pipeline559/1
  pipeline83/1 -> bus_create4/1(in1)
  pipeline84/1 -> bus_create4/2(in2)
  pipeline85/1 -> bus_create4/3(in3)
  pipeline86/1 -> bus_create4/4(in4)
  Convert24/1 -> pipeline83/1
  Convert25/1 -> pipeline84/1
  Convert22/1 -> pipeline85/1
  Convert23/1 -> pipeline86/1
  bus_create8/1(bus_out) -> pipeline511/1
  bus_create4/1(bus_out) -> pipeline664/1
  [cnt_rst] -> ten_Gbe_v2/9(debug_rst), geth_pack/1(rst)
  pipeline511/1 -> bus_create10/1(in1)
  pipeline664/1 -> bus_create10/2(in2)
  pipeline546/1 -> bus_create10/3(in3)
  pipeline577/1 -> bus_create10/4(in4)
  pipeline564/1 -> pipeline565/1
  pipeline565/1 -> edge_detect11/1, pipeline566/1
  pipeline566/1 -> Logical9/2
  geth_pack/1(dout) -> ten_Gbe_v2/2(tx_data)
  geth_pack/2(valid) -> ten_Gbe_v2/3(tx_valid)
  geth_pack/3(eof) -> ten_Gbe_v2/6(tx_end_of_frame)
  ten_Gbe_v2/12(rx_overrun) -> Delay159/1
  Delay159/1 -> ten_Gbe_v2/8(rx_overrun_ack)
  ten_Gbe_v2/1(led_up) -> gpio2/1
  ten_Gbe_v2/3(led_tx) -> gpio7/1
  dest_ip/1(in_reg) -> ten_Gbe_v2/4(tx_dest_ip)
  dest_port/1(in_reg) -> Slice15/1
  Slice15/1 -> ten_Gbe_v2/5(tx_dest_port)
  Constant110/1 -> ten_Gbe_v2/7(rx_ack)
  ten_Gbe_v2/4(tx_afull) -> Terminator98/1
  ten_Gbe_v2/5(tx_overflow) -> Terminator101/1
  ten_Gbe_v2/6(rx_data) -> Terminator102/1
  ten_Gbe_v2/7(rx_valid) -> Terminator103/1
  ten_Gbe_v2/8(rx_source_ip) -> Terminator105/1
  ten_Gbe_v2/9(rx_source_port) -> Terminator106/1
  ten_Gbe_v2/10(rx_end_of_frame) -> Terminator107/1
  ten_Gbe_v2/11(rx_bad_frame) -> Terminator108/1
  ten_Gbe_v2/13(rx_dest_ip) -> Terminator109/1
  ten_Gbe_v2/14(rx_dest_port) -> Terminator99/1
  ten_Gbe_v2/2(led_rx) -> Terminator100/1
  bus_create10/1(bus_out) -> pipeline567/1
  [cnt_rst] -> ten_Gbe_v2/1(rst)
  edge_detect11/1 -> Mux4/1, Register11/1, Register11/3, Logical9/1
  [cnt_rst] -> Register11/2
  pipeline567/1 -> Mux4/2
  Mux4/1 -> geth_pack/2(din)
  Register11/1 -> Logical25/2
  Logical9/1 -> Logical25/1
  Logical25/1 -> geth_pack/3(data_en)
  [time] -> Mux4/3
  pipeline87/1(q) -> pipeline564/1
  Constant12/1 -> pipeline577/1, pipeline546/1
  Delay106/1 -> Delay103/1
  Delay107/1 -> Delay104/1
  Delay108/1 -> Delay105/1
  Delay109/1 -> Delay101/1
  Delay110/1 -> Delay102/1
  pipeline88/1(q) -> Counter5/1
  pipeline89/1(q) -> Convert23/1, Reinterpret4/1
  pipeline90/1(q) -> spec2_0/3(we), Counter5/2
  pipeline91/1(q) -> Convert22/1, Reinterpret8/1
  pipeline92/1(q) -> spec2_1/3(we)
  pipeline93/1(q) -> Convert25/1, Reinterpret9/1
  pipeline94/1(q) -> spec2_2/3(we)
  pipeline96/1(q) -> spec2_3/3(we)
  pipeline95/1(q) -> Convert24/1, Reinterpret10/1
  pipeline97/1(q) -> Convert19/1, Reinterpret40/1
  pipeline98/1(q) -> pipeline87/1(d), spec1_0/3(we), Counter22/2
  pipeline99/1(q) -> Convert18/1, Reinterpret41/1
  pipeline100/1(q) -> spec1_1/3(we)
  pipeline102/1(q) -> spec1_2/3(we)
  pipeline101/1(q) -> Convert21/1, Reinterpret42/1
  pipeline103/1(q) -> Convert20/1, Reinterpret43/1
  pipeline104/1(q) -> spec1_3/3(we)
  pipeline105/1(q) -> Counter22/1
